simulink model "pfb_float_4k_sim"  (R2018b)
settings: Solver=VariableStepDiscrete  StartTime=0.0  StopTime=8192*2^2  RelTol=1e-3
undefined symbols (not defined in the file): fft_stages
libraries: xbsIndex_r4 x16102, casper_library_misc x27, casper_library_delays x15, casper_library_sources x4, xbsReplacements_r4 x3, xps_library x1
(part 8 of 133)

== system pfb_float_4k_sim/pfb_of ==
  out_reg : Inport
  assert_reg : xbsIndex_r4/Assert  assert_type=on  type_source=Explicitly  gui_display_data_type=Fixed-point  arith_type=Unsigned  n_bits=2  bin_pt=0  preci_type=Single  exp_width=8  frac_width=24  assert_rate=off  rate_source=Explicitly  period=1  output_port=on  block_type=assert
  cast_gw : xbsIndex_r4/Convert  gui_display_data_type=Fixed-point  arith_type=Unsigned  n_bits=32  bin_pt=0  float_type=Single  exp_bits=8  fraction_bits=24  quantization=Truncate  overflow=Wrap  en=off  latency=1  pipeline=off  dbl_ovrd=off  block_type=convert
  io_delay : xbsIndex_r4/Delay  rst=off  infoeditControl=Selection of Reset will increase slice count due to use of real FFs and instead of SRLs  en=off  latency=0  reg_retiming=on  dbl_ovrd=off  block_type=delay
  pfb_float_4k_sim_pfb_of_user_data_in : xbsIndex_r4/Gateway Out  inherit_from_input=off  hdl_port=on  rows=1  columns=1  UseAsDAC=off  DACChannel='1'  interface=None  automatic_assignment=on  address_offset=hex2dec('00')  intf_name=''  intf_description=''  timing_constraint=None  locs_specified=off  LOCs={}  io_standard={}  block_type=gatewayout
  reint1 : xbsIndex_r4/Reinterpret  force_arith_type=on  arith_type=Unsigned  force_bin_pt=on  bin_pt=0  block_type=reinterpret
  sim_out : Outport
  wires:
  io_delay/1 -> cast_gw/1
  cast_gw/1 -> pfb_float_4k_sim_pfb_of_user_data_in/1
  pfb_float_4k_sim_pfb_of_user_data_in/1 -> sim_out/1
  out_reg/1 -> assert_reg/1
  assert_reg/1 -> reint1/1
  reint1/1 -> io_delay/1

== system pfb_float_4k_sim/pfbin_sync_count ==
  out_reg : Inport
  assert_reg : xbsIndex_r4/Assert  assert_type=on  type_source=Explicitly  gui_display_data_type=Fixed-point  arith_type=Unsigned  n_bits=32  bin_pt=0  preci_type=Single  exp_width=8  frac_width=24  assert_rate=off  rate_source=Explicitly  period=1  output_port=on  block_type=assert
  cast_gw : xbsIndex_r4/Convert  gui_display_data_type=Fixed-point  arith_type=Unsigned  n_bits=32  bin_pt=0  float_type=Single  exp_bits=8  fraction_bits=24  quantization=Truncate  overflow=Wrap  en=off  latency=1  pipeline=off  dbl_ovrd=off  block_type=convert
  io_delay : xbsIndex_r4/Delay  rst=off  infoeditControl=Selection of Reset will increase slice count due to use of real FFs and instead of SRLs  en=off  latency=0  reg_retiming=on  dbl_ovrd=off  block_type=delay
  pfb_float_4k_sim_pfbin_sync_count_user_data_in : xbsIndex_r4/Gateway Out  inherit_from_input=off  hdl_port=on  rows=1  columns=1  UseAsDAC=off  DACChannel='1'  interface=None  automatic_assignment=on  address_offset=hex2dec('00')  intf_name=''  intf_description=''  timing_constraint=None  locs_specified=off  LOCs={}  io_standard={}  block_type=gatewayout
  reint1 : xbsIndex_r4/Reinterpret  force_arith_type=on  arith_type=Unsigned  force_bin_pt=on  bin_pt=0  block_type=reinterpret
  sim_out : Outport
  wires:
  io_delay/1 -> cast_gw/1
  cast_gw/1 -> pfb_float_4k_sim_pfbin_sync_count_user_data_in/1
  pfb_float_4k_sim_pfbin_sync_count_user_data_in/1 -> sim_out/1
  out_reg/1 -> assert_reg/1
  assert_reg/1 -> reint1/1
  reint1/1 -> io_delay/1

== system pfb_float_4k_sim/pfbout_sync_count ==
  out_reg : Inport
  assert_reg : xbsIndex_r4/Assert  assert_type=on  type_source=Explicitly  gui_display_data_type=Fixed-point  arith_type=Unsigned  n_bits=32  bin_pt=0  preci_type=Single  exp_width=8  frac_width=24  assert_rate=off  rate_source=Explicitly  period=1  output_port=on  block_type=assert
  cast_gw : xbsIndex_r4/Convert  gui_display_data_type=Fixed-point  arith_type=Unsigned  n_bits=32  bin_pt=0  float_type=Single  exp_bits=8  fraction_bits=24  quantization=Truncate  overflow=Wrap  en=off  latency=1  pipeline=off  dbl_ovrd=off  block_type=convert
  io_delay : xbsIndex_r4/Delay  rst=off  infoeditControl=Selection of Reset will increase slice count due to use of real FFs and instead of SRLs  en=off  latency=0  reg_retiming=on  dbl_ovrd=off  block_type=delay
  pfb_float_4k_sim_pfbout_sync_count_user_data_in : xbsIndex_r4/Gateway Out  inherit_from_input=off  hdl_port=on  rows=1  columns=1  UseAsDAC=off  DACChannel='1'  interface=None  automatic_assignment=on  address_offset=hex2dec('00')  intf_name=''  intf_description=''  timing_constraint=None  locs_specified=off  LOCs={}  io_standard={}  block_type=gatewayout
  reint1 : xbsIndex_r4/Reinterpret  force_arith_type=on  arith_type=Unsigned  force_bin_pt=on  bin_pt=0  block_type=reinterpret
  sim_out : Outport
  wires:
  io_delay/1 -> cast_gw/1
  cast_gw/1 -> pfb_float_4k_sim_pfbout_sync_count_user_data_in/1
  pfb_float_4k_sim_pfbout_sync_count_user_data_in/1 -> sim_out/1
  out_reg/1 -> assert_reg/1
  assert_reg/1 -> reint1/1
  reint1/1 -> io_delay/1

== system pfb_float_4k_sim/pipeline1 ==
  d : Inport
  Register0 : xbsIndex_r4/Register  init=0  rst=off  en=off  dbl_ovrd=off  block_type=register
      settings: block_version=11.4
  Register1 : xbsIndex_r4/Register  init=0  rst=off  en=off  dbl_ovrd=off  block_type=register
      settings: block_version=11.4
  q : Outport
  wires:
  Register0/1 -> Register1/1
  d/1 -> Register0/1
  Register1/1 -> q/1

== system pfb_float_4k_sim/pipeline10 ==
  d : Inport
  Register0 : xbsIndex_r4/Register  init=0  rst=off  en=off  dbl_ovrd=off  block_type=register
      settings: block_version=11.4
  Register1 : xbsIndex_r4/Register  init=0  rst=off  en=off  dbl_ovrd=off  block_type=register
      settings: block_version=11.4
  q : Outport
  wires:
  Register0/1 -> Register1/1
  d/1 -> Register0/1
  Register1/1 -> q/1

== system pfb_float_4k_sim/pipeline11 ==
  d : Inport
  Register0 : xbsIndex_r4/Register  init=0  rst=off  en=off  dbl_ovrd=off  block_type=register
      settings: block_version=11.4
  q : Outport
  wires:
  d/1 -> Register0/1
  Register0/1 -> q/1

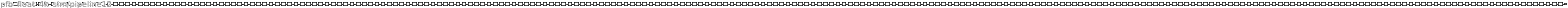
== system pfb_float_4k_sim/pipeline12 ==
  d : Inport
  Register{0..249} (250 alike) : xbsIndex_r4/Register  init=0  rst=off  en=off  dbl_ovrd=off  block_type=register
      Register0 settings: block_version=11.4
      Register1 settings: block_version=11.4
      Register2 settings: block_version=11.4
  q : Outport
  wires:
  Register0/1 -> Register1/1
  Register1/1 -> Register2/1
  Register2/1 -> Register3/1
  Register3/1 -> Register4/1
  Register4/1 -> Register5/1
  Register5/1 -> Register6/1
  Register6/1 -> Register7/1
  Register7/1 -> Register8/1
  Register8/1 -> Register9/1
  Register9/1 -> Register10/1
  Register10/1 -> Register11/1
  Register11/1 -> Register12/1
  Register12/1 -> Register13/1
  Register13/1 -> Register14/1
  Register14/1 -> Register15/1
  Register15/1 -> Register16/1
  Register16/1 -> Register17/1
  Register17/1 -> Register18/1
  Register18/1 -> Register19/1
  Register19/1 -> Register20/1
  Register20/1 -> Register21/1
  Register21/1 -> Register22/1
  Register22/1 -> Register23/1
  Register23/1 -> Register24/1
  Register24/1 -> Register25/1
  Register25/1 -> Register26/1
  Register26/1 -> Register27/1
  Register27/1 -> Register28/1
  Register28/1 -> Register29/1
  Register29/1 -> Register30/1
  Register30/1 -> Register31/1
  Register31/1 -> Register32/1
  Register32/1 -> Register33/1
  Register33/1 -> Register34/1
  Register34/1 -> Register35/1
  Register35/1 -> Register36/1
  Register36/1 -> Register37/1
  Register37/1 -> Register38/1
  Register38/1 -> Register39/1
  Register39/1 -> Register40/1
  Register40/1 -> Register41/1
  Register41/1 -> Register42/1
  Register42/1 -> Register43/1
  Register43/1 -> Register44/1
  Register44/1 -> Register45/1
  Register45/1 -> Register46/1
  Register46/1 -> Register47/1
  Register47/1 -> Register48/1
  Register48/1 -> Register49/1
  Register49/1 -> Register50/1
  Register50/1 -> Register51/1
  Register51/1 -> Register52/1
  Register52/1 -> Register53/1
  Register53/1 -> Register54/1
  Register54/1 -> Register55/1
  Register55/1 -> Register56/1
  Register56/1 -> Register57/1
  Register57/1 -> Register58/1
  Register58/1 -> Register59/1
  Register59/1 -> Register60/1
  Register60/1 -> Register61/1
  Register61/1 -> Register62/1
  Register62/1 -> Register63/1
  Register63/1 -> Register64/1
  Register64/1 -> Register65/1
  Register65/1 -> Register66/1
  Register66/1 -> Register67/1
  Register67/1 -> Register68/1
  Register68/1 -> Register69/1
  Register69/1 -> Register70/1
  Register70/1 -> Register71/1
  Register71/1 -> Register72/1
  Register72/1 -> Register73/1
  Register73/1 -> Register74/1
  Register74/1 -> Register75/1
  Register75/1 -> Register76/1
  Register76/1 -> Register77/1
  Register77/1 -> Register78/1
  Register78/1 -> Register79/1
  Register79/1 -> Register80/1
  Register80/1 -> Register81/1
  Register81/1 -> Register82/1
  Register82/1 -> Register83/1
  Register83/1 -> Register84/1
  Register84/1 -> Register85/1
  Register85/1 -> Register86/1
  Register86/1 -> Register87/1
  Register87/1 -> Register88/1
  Register88/1 -> Register89/1
  Register89/1 -> Register90/1
  Register90/1 -> Register91/1
  Register91/1 -> Register92/1
  Register92/1 -> Register93/1
  Register93/1 -> Register94/1
  Register94/1 -> Register95/1
  Register95/1 -> Register96/1
  Register96/1 -> Register97/1
  Register97/1 -> Register98/1
  Register98/1 -> Register99/1
  Register99/1 -> Register100/1
  Register100/1 -> Register101/1
  Register101/1 -> Register102/1
  Register102/1 -> Register103/1
  Register103/1 -> Register104/1
  Register104/1 -> Register105/1
  Register105/1 -> Register106/1
  Register106/1 -> Register107/1
  Register107/1 -> Register108/1
  Register108/1 -> Register109/1
  Register109/1 -> Register110/1
  Register110/1 -> Register111/1
  Register111/1 -> Register112/1
  Register112/1 -> Register113/1
  Register113/1 -> Register114/1
  Register114/1 -> Register115/1
  Register115/1 -> Register116/1
  Register116/1 -> Register117/1
  Register117/1 -> Register118/1
  Register118/1 -> Register119/1
  Register119/1 -> Register120/1
  Register120/1 -> Register121/1
  Register121/1 -> Register122/1
  Register122/1 -> Register123/1
  Register123/1 -> Register124/1
  Register124/1 -> Register125/1
  Register125/1 -> Register126/1
  Register126/1 -> Register127/1
  Register127/1 -> Register128/1
  Register128/1 -> Register129/1
  Register129/1 -> Register130/1
  Register130/1 -> Register131/1
  Register131/1 -> Register132/1
  Register132/1 -> Register133/1
  Register133/1 -> Register134/1
  Register134/1 -> Register135/1
  Register135/1 -> Register136/1
  Register136/1 -> Register137/1
  Register137/1 -> Register138/1
  Register138/1 -> Register139/1
  Register139/1 -> Register140/1
  Register140/1 -> Register141/1
  Register141/1 -> Register142/1
  Register142/1 -> Register143/1
  Register143/1 -> Register144/1
  Register144/1 -> Register145/1
  Register145/1 -> Register146/1
  Register146/1 -> Register147/1
  Register147/1 -> Register148/1
  Register148/1 -> Register149/1
  Register149/1 -> Register150/1
  Register150/1 -> Register151/1
  Register151/1 -> Register152/1
  Register152/1 -> Register153/1
  Register153/1 -> Register154/1
  Register154/1 -> Register155/1
  Register155/1 -> Register156/1
  Register156/1 -> Register157/1
  Register157/1 -> Register158/1
  Register158/1 -> Register159/1
  Register159/1 -> Register160/1
  Register160/1 -> Register161/1
  Register161/1 -> Register162/1
  Register162/1 -> Register163/1
  Register163/1 -> Register164/1
  Register164/1 -> Register165/1
  Register165/1 -> Register166/1
  Register166/1 -> Register167/1
  Register167/1 -> Register168/1
  Register168/1 -> Register169/1
  Register169/1 -> Register170/1
  Register170/1 -> Register171/1
  Register171/1 -> Register172/1
  Register172/1 -> Register173/1
  Register173/1 -> Register174/1
  Register174/1 -> Register175/1
  Register175/1 -> Register176/1
  Register176/1 -> Register177/1
  Register177/1 -> Register178/1
  Register178/1 -> Register179/1
  Register179/1 -> Register180/1
  Register180/1 -> Register181/1
  Register181/1 -> Register182/1
  Register182/1 -> Register183/1
  Register183/1 -> Register184/1
  Register184/1 -> Register185/1
  Register185/1 -> Register186/1
  Register186/1 -> Register187/1
  Register187/1 -> Register188/1
  Register188/1 -> Register189/1
  Register189/1 -> Register190/1
  Register190/1 -> Register191/1
  Register191/1 -> Register192/1
  Register192/1 -> Register193/1
  Register193/1 -> Register194/1
  Register194/1 -> Register195/1
  Register195/1 -> Register196/1
  Register196/1 -> Register197/1
  Register197/1 -> Register198/1
  Register198/1 -> Register199/1
  Register199/1 -> Register200/1
  Register200/1 -> Register201/1
  Register201/1 -> Register202/1
  Register202/1 -> Register203/1
  Register203/1 -> Register204/1
  Register204/1 -> Register205/1
  Register205/1 -> Register206/1
  Register206/1 -> Register207/1
  Register207/1 -> Register208/1
  Register208/1 -> Register209/1
  Register209/1 -> Register210/1
  Register210/1 -> Register211/1
  Register211/1 -> Register212/1
  Register212/1 -> Register213/1
  Register213/1 -> Register214/1
  Register214/1 -> Register215/1
  Register215/1 -> Register216/1
  Register216/1 -> Register217/1
  Register217/1 -> Register218/1
  Register218/1 -> Register219/1
  Register219/1 -> Register220/1
  Register220/1 -> Register221/1
  Register221/1 -> Register222/1
  Register222/1 -> Register223/1
  Register223/1 -> Register224/1
  Register224/1 -> Register225/1
  Register225/1 -> Register226/1
  Register226/1 -> Register227/1
  Register227/1 -> Register228/1
  Register228/1 -> Register229/1
  Register229/1 -> Register230/1
  Register230/1 -> Register231/1
  Register231/1 -> Register232/1
  Register232/1 -> Register233/1
  Register233/1 -> Register234/1
  Register234/1 -> Register235/1
  Register235/1 -> Register236/1
  Register236/1 -> Register237/1
  Register237/1 -> Register238/1
  Register238/1 -> Register239/1
  Register239/1 -> Register240/1
  Register240/1 -> Register241/1
  Register241/1 -> Register242/1
  Register242/1 -> Register243/1
  Register243/1 -> Register244/1
  Register244/1 -> Register245/1
  Register245/1 -> Register246/1
  Register246/1 -> Register247/1
  Register247/1 -> Register248/1
  Register248/1 -> Register249/1
  d/1 -> Register0/1
  Register249/1 -> q/1

== system pfb_float_4k_sim/pipeline13 ==
  d : Inport
  Register0 : xbsIndex_r4/Register  init=0  rst=off  en=off  dbl_ovrd=off  block_type=register
      settings: block_version=11.4
  Register1 : xbsIndex_r4/Register  init=0  rst=off  en=off  dbl_ovrd=off  block_type=register
      settings: block_version=11.4
  q : Outport
  wires:
  Register0/1 -> Register1/1
  d/1 -> Register0/1
  Register1/1 -> q/1

== system pfb_float_4k_sim/pipeline14 ==
  d : Inport
  Register0 : xbsIndex_r4/Register  init=0  rst=off  en=off  dbl_ovrd=off  block_type=register
      settings: block_version=11.4
  Register1 : xbsIndex_r4/Register  init=0  rst=off  en=off  dbl_ovrd=off  block_type=register
      settings: block_version=11.4
  q : Outport
  wires:
  Register0/1 -> Register1/1
  d/1 -> Register0/1
  Register1/1 -> q/1

== system pfb_float_4k_sim/pipeline15 ==
  d : Inport
  Register0 : xbsIndex_r4/Register  init=0  rst=off  en=off  dbl_ovrd=off  block_type=register
      settings: block_version=11.4
  q : Outport
  wires:
  d/1 -> Register0/1
  Register0/1 -> q/1

== system pfb_float_4k_sim/pipeline16 ==
  d : Inport
  Register{0..3} (4 alike) : xbsIndex_r4/Register  init=0  rst=off  en=off  dbl_ovrd=off  block_type=register
      Register0 settings: block_version=11.4
      Register1 settings: block_version=11.4
      Register2 settings: block_version=11.4
  q : Outport
  wires:
  Register0/1 -> Register1/1
  Register1/1 -> Register2/1
  Register2/1 -> Register3/1
  d/1 -> Register0/1
  Register3/1 -> q/1

== system pfb_float_4k_sim/pipeline17 ==
  d : Inport
  Register0 : xbsIndex_r4/Register  init=0  rst=off  en=off  dbl_ovrd=off  block_type=register
      settings: block_version=11.4
  Register1 : xbsIndex_r4/Register  init=0  rst=off  en=off  dbl_ovrd=off  block_type=register
      settings: block_version=11.4
  q : Outport
  wires:
  Register0/1 -> Register1/1
  d/1 -> Register0/1
  Register1/1 -> q/1

== system pfb_float_4k_sim/pipeline18 ==
  d : Inport
  Register0 : xbsIndex_r4/Register  init=0  rst=off  en=off  dbl_ovrd=off  block_type=register
      settings: block_version=11.4
  Register1 : xbsIndex_r4/Register  init=0  rst=off  en=off  dbl_ovrd=off  block_type=register
      settings: block_version=11.4
  q : Outport
  wires:
  Register0/1 -> Register1/1
  d/1 -> Register0/1
  Register1/1 -> q/1

== system pfb_float_4k_sim/pipeline180 ==
  d : Inport
  Register0 : xbsIndex_r4/Register  init=0  rst=off  en=off  dbl_ovrd=off  block_type=register
      settings: block_version=11.4
  Register1 : xbsIndex_r4/Register  init=0  rst=off  en=off  dbl_ovrd=off  block_type=register
      settings: block_version=11.4
  q : Outport
  wires:
  Register0/1 -> Register1/1
  d/1 -> Register0/1
  Register1/1 -> q/1

== system pfb_float_4k_sim/pipeline181 ==
  d : Inport
  Register0 : xbsIndex_r4/Register  init=0  rst=off  en=off  dbl_ovrd=off  block_type=register
      settings: block_version=11.4
  Register1 : xbsIndex_r4/Register  init=0  rst=off  en=off  dbl_ovrd=off  block_type=register
      settings: block_version=11.4
  q : Outport
  wires:
  Register0/1 -> Register1/1
  d/1 -> Register0/1
  Register1/1 -> q/1

== system pfb_float_4k_sim/pipeline182 ==
  d : Inport
  Register0 : xbsIndex_r4/Register  init=0  rst=off  en=off  dbl_ovrd=off  block_type=register
      settings: block_version=11.4
  Register1 : xbsIndex_r4/Register  init=0  rst=off  en=off  dbl_ovrd=off  block_type=register
      settings: block_version=11.4
  q : Outport
  wires:
  Register0/1 -> Register1/1
  d/1 -> Register0/1
  Register1/1 -> q/1

== system pfb_float_4k_sim/pipeline19 ==
  d : Inport
  Register0 : xbsIndex_r4/Register  init=0  rst=off  en=off  dbl_ovrd=off  block_type=register
      settings: block_version=11.4
  Register1 : xbsIndex_r4/Register  init=0  rst=off  en=off  dbl_ovrd=off  block_type=register
      settings: block_version=11.4
  q : Outport
  wires:
  Register0/1 -> Register1/1
  d/1 -> Register0/1
  Register1/1 -> q/1

== system pfb_float_4k_sim/pipeline198 ==
  d : Inport
  Register0 : xbsIndex_r4/Register  init=0  rst=off  en=off  dbl_ovrd=off  block_type=register
      settings: block_version=11.4
  Register1 : xbsIndex_r4/Register  init=0  rst=off  en=off  dbl_ovrd=off  block_type=register
      settings: block_version=11.4
  q : Outport
  wires:
  Register0/1 -> Register1/1
  d/1 -> Register0/1
  Register1/1 -> q/1

== system pfb_float_4k_sim/pipeline2 ==
  d : Inport
  Register0 : xbsIndex_r4/Register  init=0  rst=off  en=off  dbl_ovrd=off  block_type=register
      settings: block_version=11.4
  Register1 : xbsIndex_r4/Register  init=0  rst=off  en=off  dbl_ovrd=off  block_type=register
      settings: block_version=11.4
  q : Outport
  wires:
  Register0/1 -> Register1/1
  d/1 -> Register0/1
  Register1/1 -> q/1

== system pfb_float_4k_sim/pipeline20 ==
  d : Inport
  Register0 : xbsIndex_r4/Register  init=0  rst=off  en=off  dbl_ovrd=off  block_type=register
      settings: block_version=11.4
  Register1 : xbsIndex_r4/Register  init=0  rst=off  en=off  dbl_ovrd=off  block_type=register
      settings: block_version=11.4
  q : Outport
  wires:
  Register0/1 -> Register1/1
  d/1 -> Register0/1
  Register1/1 -> q/1

== system pfb_float_4k_sim/pipeline21 ==
  d : Inport
  Register0 : xbsIndex_r4/Register  init=0  rst=off  en=off  dbl_ovrd=off  block_type=register
      settings: block_version=11.4
  Register1 : xbsIndex_r4/Register  init=0  rst=off  en=off  dbl_ovrd=off  block_type=register
      settings: block_version=11.4
  q : Outport
  wires:
  Register0/1 -> Register1/1
  d/1 -> Register0/1
  Register1/1 -> q/1

== system pfb_float_4k_sim/pipeline22 ==
  d : Inport
  Register0 : xbsIndex_r4/Register  init=0  rst=off  en=off  dbl_ovrd=off  block_type=register
      settings: block_version=11.4
  Register1 : xbsIndex_r4/Register  init=0  rst=off  en=off  dbl_ovrd=off  block_type=register
      settings: block_version=11.4
  q : Outport
  wires:
  Register0/1 -> Register1/1
  d/1 -> Register0/1
  Register1/1 -> q/1

== system pfb_float_4k_sim/pipeline23 ==
  d : Inport
  Register0 : xbsIndex_r4/Register  init=0  rst=off  en=off  dbl_ovrd=off  block_type=register
      settings: block_version=11.4
  Register1 : xbsIndex_r4/Register  init=0  rst=off  en=off  dbl_ovrd=off  block_type=register
      settings: block_version=11.4
  q : Outport
  wires:
  Register0/1 -> Register1/1
  d/1 -> Register0/1
  Register1/1 -> q/1

== system pfb_float_4k_sim/pipeline24 ==
  d : Inport
  Register0 : xbsIndex_r4/Register  init=0  rst=off  en=off  dbl_ovrd=off  block_type=register
      settings: block_version=11.4
  Register1 : xbsIndex_r4/Register  init=0  rst=off  en=off  dbl_ovrd=off  block_type=register
      settings: block_version=11.4
  q : Outport
  wires:
  Register0/1 -> Register1/1
  d/1 -> Register0/1
  Register1/1 -> q/1

== system pfb_float_4k_sim/pipeline25 ==
  d : Inport
  Register0 : xbsIndex_r4/Register  init=0  rst=off  en=off  dbl_ovrd=off  block_type=register
      settings: block_version=11.4
  Register1 : xbsIndex_r4/Register  init=0  rst=off  en=off  dbl_ovrd=off  block_type=register
      settings: block_version=11.4
  q : Outport
  wires:
  Register0/1 -> Register1/1
  d/1 -> Register0/1
  Register1/1 -> q/1

== system pfb_float_4k_sim/pipeline26 ==
  d : Inport
  Register0 : xbsIndex_r4/Register  init=0  rst=off  en=off  dbl_ovrd=off  block_type=register
      settings: block_version=11.4
  Register1 : xbsIndex_r4/Register  init=0  rst=off  en=off  dbl_ovrd=off  block_type=register
      settings: block_version=11.4
  q : Outport
  wires:
  Register0/1 -> Register1/1
  d/1 -> Register0/1
  Register1/1 -> q/1

== system pfb_float_4k_sim/pipeline27 ==
  d : Inport
  Register0 : xbsIndex_r4/Register  init=0  rst=off  en=off  dbl_ovrd=off  block_type=register
      settings: block_version=11.4
  Register1 : xbsIndex_r4/Register  init=0  rst=off  en=off  dbl_ovrd=off  block_type=register
      settings: block_version=11.4
  q : Outport
  wires:
  Register0/1 -> Register1/1
  d/1 -> Register0/1
  Register1/1 -> q/1

== system pfb_float_4k_sim/pipeline28 ==
  d : Inport
  Register0 : xbsIndex_r4/Register  init=0  rst=off  en=off  dbl_ovrd=off  block_type=register
      settings: block_version=11.4
  Register1 : xbsIndex_r4/Register  init=0  rst=off  en=off  dbl_ovrd=off  block_type=register
      settings: block_version=11.4
  q : Outport
  wires:
  Register0/1 -> Register1/1
  d/1 -> Register0/1
  Register1/1 -> q/1

== system pfb_float_4k_sim/pipeline29 ==
  d : Inport
  Register0 : xbsIndex_r4/Register  init=0  rst=off  en=off  dbl_ovrd=off  block_type=register
      settings: block_version=11.4
  Register1 : xbsIndex_r4/Register  init=0  rst=off  en=off  dbl_ovrd=off  block_type=register
      settings: block_version=11.4
  q : Outport
  wires:
  Register0/1 -> Register1/1
  d/1 -> Register0/1
  Register1/1 -> q/1

== system pfb_float_4k_sim/pipeline3 ==
  d : Inport
  Register0 : xbsIndex_r4/Register  init=0  rst=off  en=off  dbl_ovrd=off  block_type=register
      settings: block_version=11.4
  Register1 : xbsIndex_r4/Register  init=0  rst=off  en=off  dbl_ovrd=off  block_type=register
      settings: block_version=11.4
  q : Outport
  wires:
  Register0/1 -> Register1/1
  d/1 -> Register0/1
  Register1/1 -> q/1

== system pfb_float_4k_sim/pipeline30 ==
  d : Inport
  Register0 : xbsIndex_r4/Register  init=0  rst=off  en=off  dbl_ovrd=off  block_type=register
      settings: block_version=11.4
  Register1 : xbsIndex_r4/Register  init=0  rst=off  en=off  dbl_ovrd=off  block_type=register
      settings: block_version=11.4
  q : Outport
  wires:
  Register0/1 -> Register1/1
  d/1 -> Register0/1
  Register1/1 -> q/1

== system pfb_float_4k_sim/pipeline31 ==
  d : Inport
  Register0 : xbsIndex_r4/Register  init=0  rst=off  en=off  dbl_ovrd=off  block_type=register
      settings: block_version=11.4
  Register1 : xbsIndex_r4/Register  init=0  rst=off  en=off  dbl_ovrd=off  block_type=register
      settings: block_version=11.4
  q : Outport
  wires:
  Register0/1 -> Register1/1
  d/1 -> Register0/1
  Register1/1 -> q/1

== system pfb_float_4k_sim/pipeline32 ==
  d : Inport
  Register0 : xbsIndex_r4/Register  init=0  rst=off  en=off  dbl_ovrd=off  block_type=register
      settings: block_version=11.4
  Register1 : xbsIndex_r4/Register  init=0  rst=off  en=off  dbl_ovrd=off  block_type=register
      settings: block_version=11.4
  q : Outport
  wires:
  Register0/1 -> Register1/1
  d/1 -> Register0/1
  Register1/1 -> q/1

== system pfb_float_4k_sim/pipeline33 ==
  d : Inport
  Register0 : xbsIndex_r4/Register  init=0  rst=off  en=off  dbl_ovrd=off  block_type=register
      settings: block_version=11.4
  Register1 : xbsIndex_r4/Register  init=0  rst=off  en=off  dbl_ovrd=off  block_type=register
      settings: block_version=11.4
  q : Outport
  wires:
  Register0/1 -> Register1/1
  d/1 -> Register0/1
  Register1/1 -> q/1

== system pfb_float_4k_sim/pipeline34 ==
  d : Inport
  Register0 : xbsIndex_r4/Register  init=0  rst=off  en=off  dbl_ovrd=off  block_type=register
      settings: block_version=11.4
  Register1 : xbsIndex_r4/Register  init=0  rst=off  en=off  dbl_ovrd=off  block_type=register
      settings: block_version=11.4
  q : Outport
  wires:
  Register0/1 -> Register1/1
  d/1 -> Register0/1
  Register1/1 -> q/1

== system pfb_float_4k_sim/pipeline35 ==
  d : Inport
  Register0 : xbsIndex_r4/Register  init=0  rst=off  en=off  dbl_ovrd=off  block_type=register
      settings: block_version=11.4
  Register1 : xbsIndex_r4/Register  init=0  rst=off  en=off  dbl_ovrd=off  block_type=register
      settings: block_version=11.4
  q : Outport
  wires:
  Register0/1 -> Register1/1
  d/1 -> Register0/1
  Register1/1 -> q/1

== system pfb_float_4k_sim/pipeline36 ==
  d : Inport
  Register0 : xbsIndex_r4/Register  init=0  rst=off  en=off  dbl_ovrd=off  block_type=register
      settings: block_version=11.4
  Register1 : xbsIndex_r4/Register  init=0  rst=off  en=off  dbl_ovrd=off  block_type=register
      settings: block_version=11.4
  q : Outport
  wires:
  Register0/1 -> Register1/1
  d/1 -> Register0/1
  Register1/1 -> q/1

== system pfb_float_4k_sim/pipeline37 ==
  d : Inport
  Register0 : xbsIndex_r4/Register  init=0  rst=off  en=off  dbl_ovrd=off  block_type=register
      settings: block_version=11.4
  Register1 : xbsIndex_r4/Register  init=0  rst=off  en=off  dbl_ovrd=off  block_type=register
      settings: block_version=11.4
  q : Outport
  wires:
  Register0/1 -> Register1/1
  d/1 -> Register0/1
  Register1/1 -> q/1

== system pfb_float_4k_sim/pipeline38 ==
  d : Inport
  Register0 : xbsIndex_r4/Register  init=0  rst=off  en=off  dbl_ovrd=off  block_type=register
      settings: block_version=11.4
  q : Outport
  wires:
  d/1 -> Register0/1
  Register0/1 -> q/1

== system pfb_float_4k_sim/pipeline39 ==
  d : Inport
  Register0 : xbsIndex_r4/Register  init=0  rst=off  en=off  dbl_ovrd=off  block_type=register
      settings: block_version=11.4
  q : Outport
  wires:
  d/1 -> Register0/1
  Register0/1 -> q/1

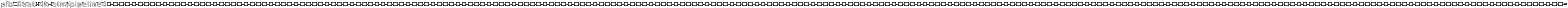
== system pfb_float_4k_sim/pipeline4 ==
  d : Inport
  Register{0..249} (250 alike) : xbsIndex_r4/Register  init=0  rst=off  en=off  dbl_ovrd=off  block_type=register
      Register0 settings: block_version=11.4
      Register1 settings: block_version=11.4
      Register2 settings: block_version=11.4
  q : Outport
  wires:
  Register0/1 -> Register1/1
  Register1/1 -> Register2/1
  Register2/1 -> Register3/1
  Register3/1 -> Register4/1
  Register4/1 -> Register5/1
  Register5/1 -> Register6/1
  Register6/1 -> Register7/1
  Register7/1 -> Register8/1
  Register8/1 -> Register9/1
  Register9/1 -> Register10/1
  Register10/1 -> Register11/1
  Register11/1 -> Register12/1
  Register12/1 -> Register13/1
  Register13/1 -> Register14/1
  Register14/1 -> Register15/1
  Register15/1 -> Register16/1
  Register16/1 -> Register17/1
  Register17/1 -> Register18/1
  Register18/1 -> Register19/1
  Register19/1 -> Register20/1
  Register20/1 -> Register21/1
  Register21/1 -> Register22/1
  Register22/1 -> Register23/1
  Register23/1 -> Register24/1
  Register24/1 -> Register25/1
  Register25/1 -> Register26/1
  Register26/1 -> Register27/1
  Register27/1 -> Register28/1
  Register28/1 -> Register29/1
  Register29/1 -> Register30/1
  Register30/1 -> Register31/1
  Register31/1 -> Register32/1
  Register32/1 -> Register33/1
  Register33/1 -> Register34/1
  Register34/1 -> Register35/1
  Register35/1 -> Register36/1
  Register36/1 -> Register37/1
  Register37/1 -> Register38/1
  Register38/1 -> Register39/1
  Register39/1 -> Register40/1
  Register40/1 -> Register41/1
  Register41/1 -> Register42/1
  Register42/1 -> Register43/1
  Register43/1 -> Register44/1
  Register44/1 -> Register45/1
  Register45/1 -> Register46/1
  Register46/1 -> Register47/1
  Register47/1 -> Register48/1
  Register48/1 -> Register49/1
  Register49/1 -> Register50/1
  Register50/1 -> Register51/1
  Register51/1 -> Register52/1
  Register52/1 -> Register53/1
  Register53/1 -> Register54/1
  Register54/1 -> Register55/1
  Register55/1 -> Register56/1
  Register56/1 -> Register57/1
  Register57/1 -> Register58/1
  Register58/1 -> Register59/1
  Register59/1 -> Register60/1
  Register60/1 -> Register61/1
  Register61/1 -> Register62/1
  Register62/1 -> Register63/1
  Register63/1 -> Register64/1
  Register64/1 -> Register65/1
  Register65/1 -> Register66/1
  Register66/1 -> Register67/1
  Register67/1 -> Register68/1
  Register68/1 -> Register69/1
  Register69/1 -> Register70/1
  Register70/1 -> Register71/1
  Register71/1 -> Register72/1
  Register72/1 -> Register73/1
  Register73/1 -> Register74/1
  Register74/1 -> Register75/1
  Register75/1 -> Register76/1
  Register76/1 -> Register77/1
  Register77/1 -> Register78/1
  Register78/1 -> Register79/1
  Register79/1 -> Register80/1
  Register80/1 -> Register81/1
  Register81/1 -> Register82/1
  Register82/1 -> Register83/1
  Register83/1 -> Register84/1
  Register84/1 -> Register85/1
  Register85/1 -> Register86/1
  Register86/1 -> Register87/1
  Register87/1 -> Register88/1
  Register88/1 -> Register89/1
  Register89/1 -> Register90/1
  Register90/1 -> Register91/1
  Register91/1 -> Register92/1
  Register92/1 -> Register93/1
  Register93/1 -> Register94/1
  Register94/1 -> Register95/1
  Register95/1 -> Register96/1
  Register96/1 -> Register97/1
  Register97/1 -> Register98/1
  Register98/1 -> Register99/1
  Register99/1 -> Register100/1
  Register100/1 -> Register101/1
  Register101/1 -> Register102/1
  Register102/1 -> Register103/1
  Register103/1 -> Register104/1
  Register104/1 -> Register105/1
  Register105/1 -> Register106/1
  Register106/1 -> Register107/1
  Register107/1 -> Register108/1
  Register108/1 -> Register109/1
  Register109/1 -> Register110/1
  Register110/1 -> Register111/1
  Register111/1 -> Register112/1
  Register112/1 -> Register113/1
  Register113/1 -> Register114/1
  Register114/1 -> Register115/1
  Register115/1 -> Register116/1
  Register116/1 -> Register117/1
  Register117/1 -> Register118/1
  Register118/1 -> Register119/1
  Register119/1 -> Register120/1
  Register120/1 -> Register121/1
  Register121/1 -> Register122/1
  Register122/1 -> Register123/1
  Register123/1 -> Register124/1
  Register124/1 -> Register125/1
  Register125/1 -> Register126/1
  Register126/1 -> Register127/1
  Register127/1 -> Register128/1
  Register128/1 -> Register129/1
  Register129/1 -> Register130/1
  Register130/1 -> Register131/1
  Register131/1 -> Register132/1
  Register132/1 -> Register133/1
  Register133/1 -> Register134/1
  Register134/1 -> Register135/1
  Register135/1 -> Register136/1
  Register136/1 -> Register137/1
  Register137/1 -> Register138/1
  Register138/1 -> Register139/1
  Register139/1 -> Register140/1
  Register140/1 -> Register141/1
  Register141/1 -> Register142/1
  Register142/1 -> Register143/1
  Register143/1 -> Register144/1
  Register144/1 -> Register145/1
  Register145/1 -> Register146/1
  Register146/1 -> Register147/1
  Register147/1 -> Register148/1
  Register148/1 -> Register149/1
  Register149/1 -> Register150/1
  Register150/1 -> Register151/1
  Register151/1 -> Register152/1
  Register152/1 -> Register153/1
  Register153/1 -> Register154/1
  Register154/1 -> Register155/1
  Register155/1 -> Register156/1
  Register156/1 -> Register157/1
  Register157/1 -> Register158/1
  Register158/1 -> Register159/1
  Register159/1 -> Register160/1
  Register160/1 -> Register161/1
  Register161/1 -> Register162/1
  Register162/1 -> Register163/1
  Register163/1 -> Register164/1
  Register164/1 -> Register165/1
  Register165/1 -> Register166/1
  Register166/1 -> Register167/1
  Register167/1 -> Register168/1
  Register168/1 -> Register169/1
  Register169/1 -> Register170/1
  Register170/1 -> Register171/1
  Register171/1 -> Register172/1
  Register172/1 -> Register173/1
  Register173/1 -> Register174/1
  Register174/1 -> Register175/1
  Register175/1 -> Register176/1
  Register176/1 -> Register177/1
  Register177/1 -> Register178/1
  Register178/1 -> Register179/1
  Register179/1 -> Register180/1
  Register180/1 -> Register181/1
  Register181/1 -> Register182/1
  Register182/1 -> Register183/1
  Register183/1 -> Register184/1
  Register184/1 -> Register185/1
  Register185/1 -> Register186/1
  Register186/1 -> Register187/1
  Register187/1 -> Register188/1
  Register188/1 -> Register189/1
  Register189/1 -> Register190/1
  Register190/1 -> Register191/1
  Register191/1 -> Register192/1
  Register192/1 -> Register193/1
  Register193/1 -> Register194/1
  Register194/1 -> Register195/1
  Register195/1 -> Register196/1
  Register196/1 -> Register197/1
  Register197/1 -> Register198/1
  Register198/1 -> Register199/1
  Register199/1 -> Register200/1
  Register200/1 -> Register201/1
  Register201/1 -> Register202/1
  Register202/1 -> Register203/1
  Register203/1 -> Register204/1
  Register204/1 -> Register205/1
  Register205/1 -> Register206/1
  Register206/1 -> Register207/1
  Register207/1 -> Register208/1
  Register208/1 -> Register209/1
  Register209/1 -> Register210/1
  Register210/1 -> Register211/1
  Register211/1 -> Register212/1
  Register212/1 -> Register213/1
  Register213/1 -> Register214/1
  Register214/1 -> Register215/1
  Register215/1 -> Register216/1
  Register216/1 -> Register217/1
  Register217/1 -> Register218/1
  Register218/1 -> Register219/1
  Register219/1 -> Register220/1
  Register220/1 -> Register221/1
  Register221/1 -> Register222/1
  Register222/1 -> Register223/1
  Register223/1 -> Register224/1
  Register224/1 -> Register225/1
  Register225/1 -> Register226/1
  Register226/1 -> Register227/1
  Register227/1 -> Register228/1
  Register228/1 -> Register229/1
  Register229/1 -> Register230/1
  Register230/1 -> Register231/1
  Register231/1 -> Register232/1
  Register232/1 -> Register233/1
  Register233/1 -> Register234/1
  Register234/1 -> Register235/1
  Register235/1 -> Register236/1
  Register236/1 -> Register237/1
  Register237/1 -> Register238/1
  Register238/1 -> Register239/1
  Register239/1 -> Register240/1
  Register240/1 -> Register241/1
  Register241/1 -> Register242/1
  Register242/1 -> Register243/1
  Register243/1 -> Register244/1
  Register244/1 -> Register245/1
  Register245/1 -> Register246/1
  Register246/1 -> Register247/1
  Register247/1 -> Register248/1
  Register248/1 -> Register249/1
  d/1 -> Register0/1
  Register249/1 -> q/1

== system pfb_float_4k_sim/pipeline40 ==
  d : Inport
  Register0 : xbsIndex_r4/Register  init=0  rst=off  en=off  dbl_ovrd=off  block_type=register
      settings: block_version=11.4
  q : Outport
  wires:
  d/1 -> Register0/1
  Register0/1 -> q/1

== system pfb_float_4k_sim/pipeline41 ==
  d : Inport
  Register0 : xbsIndex_r4/Register  init=0  rst=off  en=off  dbl_ovrd=off  block_type=register
      settings: block_version=11.4
  Register1 : xbsIndex_r4/Register  init=0  rst=off  en=off  dbl_ovrd=off  block_type=register
      settings: block_version=11.4
  q : Outport
  wires:
  Register0/1 -> Register1/1
  d/1 -> Register0/1
  Register1/1 -> q/1

== system pfb_float_4k_sim/pipeline42 ==
  d : Inport
  Register0 : xbsIndex_r4/Register  init=0  rst=off  en=off  dbl_ovrd=off  block_type=register
      settings: block_version=11.4
  q : Outport
  wires:
  d/1 -> Register0/1
  Register0/1 -> q/1

== system pfb_float_4k_sim/pipeline43 ==
  d : Inport
  Register0 : xbsIndex_r4/Register  init=0  rst=off  en=off  dbl_ovrd=off  block_type=register
      settings: block_version=11.4
  q : Outport
  wires:
  d/1 -> Register0/1
  Register0/1 -> q/1

== system pfb_float_4k_sim/pipeline44 ==
  d : Inport
  Register0 : xbsIndex_r4/Register  init=0  rst=off  en=off  dbl_ovrd=off  block_type=register
      settings: block_version=11.4
  Register1 : xbsIndex_r4/Register  init=0  rst=off  en=off  dbl_ovrd=off  block_type=register
      settings: block_version=11.4
  q : Outport
  wires:
  Register0/1 -> Register1/1
  d/1 -> Register0/1
  Register1/1 -> q/1

== system pfb_float_4k_sim/pipeline45 ==
  d : Inport
  Register0 : xbsIndex_r4/Register  init=0  rst=off  en=off  dbl_ovrd=off  block_type=register
      settings: block_version=11.4
  Register1 : xbsIndex_r4/Register  init=0  rst=off  en=off  dbl_ovrd=off  block_type=register
      settings: block_version=11.4
  q : Outport
  wires:
  Register0/1 -> Register1/1
  d/1 -> Register0/1
  Register1/1 -> q/1

== system pfb_float_4k_sim/pipeline46 ==
  d : Inport
  Register0 : xbsIndex_r4/Register  init=0  rst=off  en=off  dbl_ovrd=off  block_type=register
      settings: block_version=11.4
  q : Outport
  wires:
  d/1 -> Register0/1
  Register0/1 -> q/1

== system pfb_float_4k_sim/pipeline47 ==
  d : Inport
  Register0 : xbsIndex_r4/Register  init=0  rst=off  en=off  dbl_ovrd=off  block_type=register
      settings: block_version=11.4
  Register1 : xbsIndex_r4/Register  init=0  rst=off  en=off  dbl_ovrd=off  block_type=register
      settings: block_version=11.4
  q : Outport
  wires:
  Register0/1 -> Register1/1
  d/1 -> Register0/1
  Register1/1 -> q/1

== system pfb_float_4k_sim/pipeline48 ==
  d : Inport
  Register0 : xbsIndex_r4/Register  init=0  rst=off  en=off  dbl_ovrd=off  block_type=register
      settings: block_version=11.4
  Register1 : xbsIndex_r4/Register  init=0  rst=off  en=off  dbl_ovrd=off  block_type=register
      settings: block_version=11.4
  q : Outport
  wires:
  Register0/1 -> Register1/1
  d/1 -> Register0/1
  Register1/1 -> q/1

== system pfb_float_4k_sim/pipeline49 ==
  d : Inport
  Register0 : xbsIndex_r4/Register  init=0  rst=off  en=off  dbl_ovrd=off  block_type=register
      settings: block_version=11.4
  Register1 : xbsIndex_r4/Register  init=0  rst=off  en=off  dbl_ovrd=off  block_type=register
      settings: block_version=11.4
  q : Outport
  wires:
  Register0/1 -> Register1/1
  d/1 -> Register0/1
  Register1/1 -> q/1

== system pfb_float_4k_sim/pipeline5 ==
  d : Inport
  Register0 : xbsIndex_r4/Register  init=0  rst=off  en=off  dbl_ovrd=off  block_type=register
      settings: block_version=11.4
  Register1 : xbsIndex_r4/Register  init=0  rst=off  en=off  dbl_ovrd=off  block_type=register
      settings: block_version=11.4
  q : Outport
  wires:
  Register0/1 -> Register1/1
  d/1 -> Register0/1
  Register1/1 -> q/1

== system pfb_float_4k_sim/pipeline50 ==
  d : Inport
  Register0 : xbsIndex_r4/Register  init=0  rst=off  en=off  dbl_ovrd=off  block_type=register
      settings: block_version=11.4
  Register1 : xbsIndex_r4/Register  init=0  rst=off  en=off  dbl_ovrd=off  block_type=register
      settings: block_version=11.4
  q : Outport
  wires:
  Register0/1 -> Register1/1
  d/1 -> Register0/1
  Register1/1 -> q/1

== system pfb_float_4k_sim/pipeline51 ==
  d : Inport
  Register0 : xbsIndex_r4/Register  init=0  rst=off  en=off  dbl_ovrd=off  block_type=register
      settings: block_version=11.4
  Register1 : xbsIndex_r4/Register  init=0  rst=off  en=off  dbl_ovrd=off  block_type=register
      settings: block_version=11.4
  q : Outport
  wires:
  Register0/1 -> Register1/1
  d/1 -> Register0/1
  Register1/1 -> q/1

== system pfb_float_4k_sim/pipeline52 ==
  d : Inport
  Register0 : xbsIndex_r4/Register  init=0  rst=off  en=off  dbl_ovrd=off  block_type=register
      settings: block_version=11.4
  Register1 : xbsIndex_r4/Register  init=0  rst=off  en=off  dbl_ovrd=off  block_type=register
      settings: block_version=11.4
  q : Outport
  wires:
  Register0/1 -> Register1/1
  d/1 -> Register0/1
  Register1/1 -> q/1

== system pfb_float_4k_sim/pipeline53 ==
  d : Inport
  Register0 : xbsIndex_r4/Register  init=0  rst=off  en=off  dbl_ovrd=off  block_type=register
      settings: block_version=11.4
  q : Outport
  wires:
  d/1 -> Register0/1
  Register0/1 -> q/1

== system pfb_float_4k_sim/pipeline54 ==
  d : Inport
  Register0 : xbsIndex_r4/Register  init=0  rst=off  en=off  dbl_ovrd=off  block_type=register
      settings: block_version=11.4
  Register1 : xbsIndex_r4/Register  init=0  rst=off  en=off  dbl_ovrd=off  block_type=register
      settings: block_version=11.4
  q : Outport
  wires:
  Register0/1 -> Register1/1
  d/1 -> Register0/1
  Register1/1 -> q/1

== system pfb_float_4k_sim/pipeline55 ==
  d : Inport
  Register{0..3} (4 alike) : xbsIndex_r4/Register  init=0  rst=off  en=off  dbl_ovrd=off  block_type=register
      Register0 settings: block_version=11.4
      Register1 settings: block_version=11.4
      Register2 settings: block_version=11.4
  q : Outport
  wires:
  Register0/1 -> Register1/1
  Register1/1 -> Register2/1
  Register2/1 -> Register3/1
  d/1 -> Register0/1
  Register3/1 -> q/1

== system pfb_float_4k_sim/pipeline56 ==
  d : Inport
  Register{0..3} (4 alike) : xbsIndex_r4/Register  init=0  rst=off  en=off  dbl_ovrd=off  block_type=register
      Register0 settings: block_version=11.4
      Register1 settings: block_version=11.4
      Register2 settings: block_version=11.4
  q : Outport
  wires:
  Register0/1 -> Register1/1
  Register1/1 -> Register2/1
  Register2/1 -> Register3/1
  d/1 -> Register0/1
  Register3/1 -> q/1

== system pfb_float_4k_sim/pipeline57 ==
  d : Inport
  Register0 : xbsIndex_r4/Register  init=0  rst=off  en=off  dbl_ovrd=off  block_type=register
      settings: block_version=11.4
  Register1 : xbsIndex_r4/Register  init=0  rst=off  en=off  dbl_ovrd=off  block_type=register
      settings: block_version=11.4
  q : Outport
  wires:
  Register0/1 -> Register1/1
  d/1 -> Register0/1
  Register1/1 -> q/1

== system pfb_float_4k_sim/pipeline58 ==
  d : Inport
  Register0 : xbsIndex_r4/Register  init=0  rst=off  en=off  dbl_ovrd=off  block_type=register
      settings: block_version=11.4
  Register1 : xbsIndex_r4/Register  init=0  rst=off  en=off  dbl_ovrd=off  block_type=register
      settings: block_version=11.4
  q : Outport
  wires:
  Register0/1 -> Register1/1
  d/1 -> Register0/1
  Register1/1 -> q/1

== system pfb_float_4k_sim/pipeline59 ==
  d : Inport
  Register{0..3} (4 alike) : xbsIndex_r4/Register  init=0  rst=off  en=off  dbl_ovrd=off  block_type=register
      Register0 settings: block_version=11.4
      Register1 settings: block_version=11.4
      Register2 settings: block_version=11.4
  q : Outport
  wires:
  Register0/1 -> Register1/1
  Register1/1 -> Register2/1
  Register2/1 -> Register3/1
  d/1 -> Register0/1
  Register3/1 -> q/1

== system pfb_float_4k_sim/pipeline6 ==
  d : Inport
  Register0 : xbsIndex_r4/Register  init=0  rst=off  en=off  dbl_ovrd=off  block_type=register
      settings: block_version=11.4
  Register1 : xbsIndex_r4/Register  init=0  rst=off  en=off  dbl_ovrd=off  block_type=register
      settings: block_version=11.4
  q : Outport
  wires:
  Register0/1 -> Register1/1
  d/1 -> Register0/1
  Register1/1 -> q/1

== system pfb_float_4k_sim/pipeline60 ==
  d : Inport
  Register0 : xbsIndex_r4/Register  init=0  rst=off  en=off  dbl_ovrd=off  block_type=register
      settings: block_version=11.4
  Register1 : xbsIndex_r4/Register  init=0  rst=off  en=off  dbl_ovrd=off  block_type=register
      settings: block_version=11.4
  q : Outport
  wires:
  Register0/1 -> Register1/1
  d/1 -> Register0/1
  Register1/1 -> q/1

== system pfb_float_4k_sim/pipeline61 ==
  d : Inport
  Register{0..3} (4 alike) : xbsIndex_r4/Register  init=0  rst=off  en=off  dbl_ovrd=off  block_type=register
      Register0 settings: block_version=11.4
      Register1 settings: block_version=11.4
      Register2 settings: block_version=11.4
  q : Outport
  wires:
  Register0/1 -> Register1/1
  Register1/1 -> Register2/1
  Register2/1 -> Register3/1
  d/1 -> Register0/1
  Register3/1 -> q/1

== system pfb_float_4k_sim/pipeline62 ==
  d : Inport
  Register{0..3} (4 alike) : xbsIndex_r4/Register  init=0  rst=off  en=off  dbl_ovrd=off  block_type=register
      Register0 settings: block_version=11.4
      Register1 settings: block_version=11.4
      Register2 settings: block_version=11.4
  q : Outport
  wires:
  Register0/1 -> Register1/1
  Register1/1 -> Register2/1
  Register2/1 -> Register3/1
  d/1 -> Register0/1
  Register3/1 -> q/1

== system pfb_float_4k_sim/pipeline63 ==
  d : Inport
  Register0 : xbsIndex_r4/Register  init=0  rst=off  en=off  dbl_ovrd=off  block_type=register
      settings: block_version=11.4
  Register1 : xbsIndex_r4/Register  init=0  rst=off  en=off  dbl_ovrd=off  block_type=register
      settings: block_version=11.4
  q : Outport
  wires:
  Register0/1 -> Register1/1
  d/1 -> Register0/1
  Register1/1 -> q/1

== system pfb_float_4k_sim/pipeline64 ==
  d : Inport
  Register0 : xbsIndex_r4/Register  init=0  rst=off  en=off  dbl_ovrd=off  block_type=register
      settings: block_version=11.4
  Register1 : xbsIndex_r4/Register  init=0  rst=off  en=off  dbl_ovrd=off  block_type=register
      settings: block_version=11.4
  q : Outport
  wires:
  Register0/1 -> Register1/1
  d/1 -> Register0/1
  Register1/1 -> q/1

== system pfb_float_4k_sim/pipeline65 ==
  d : Inport
  Register0 : xbsIndex_r4/Register  init=0  rst=off  en=off  dbl_ovrd=off  block_type=register
      settings: block_version=11.4
  Register1 : xbsIndex_r4/Register  init=0  rst=off  en=off  dbl_ovrd=off  block_type=register
      settings: block_version=11.4
  q : Outport
  wires:
  Register0/1 -> Register1/1
  d/1 -> Register0/1
  Register1/1 -> q/1

== system pfb_float_4k_sim/pipeline66 ==
  d : Inport
  Register0 : xbsIndex_r4/Register  init=0  rst=off  en=off  dbl_ovrd=off  block_type=register
      settings: block_version=11.4
  Register1 : xbsIndex_r4/Register  init=0  rst=off  en=off  dbl_ovrd=off  block_type=register
      settings: block_version=11.4
  q : Outport
  wires:
  Register0/1 -> Register1/1
  d/1 -> Register0/1
  Register1/1 -> q/1

== system pfb_float_4k_sim/pipeline67 ==
  d : Inport
  Register0 : xbsIndex_r4/Register  init=0  rst=off  en=off  dbl_ovrd=off  block_type=register
      settings: block_version=11.4
  Register1 : xbsIndex_r4/Register  init=0  rst=off  en=off  dbl_ovrd=off  block_type=register
      settings: block_version=11.4
  q : Outport
  wires:
  Register0/1 -> Register1/1
  d/1 -> Register0/1
  Register1/1 -> q/1

== system pfb_float_4k_sim/pipeline68 ==
  d : Inport
  Register0 : xbsIndex_r4/Register  init=0  rst=off  en=off  dbl_ovrd=off  block_type=register
      settings: block_version=11.4
  Register1 : xbsIndex_r4/Register  init=0  rst=off  en=off  dbl_ovrd=off  block_type=register
      settings: block_version=11.4
  q : Outport
  wires:
  Register0/1 -> Register1/1
  d/1 -> Register0/1
  Register1/1 -> q/1

== system pfb_float_4k_sim/pipeline69 ==
  d : Inport
  Register0 : xbsIndex_r4/Register  init=0  rst=off  en=off  dbl_ovrd=off  block_type=register
      settings: block_version=11.4
  Register1 : xbsIndex_r4/Register  init=0  rst=off  en=off  dbl_ovrd=off  block_type=register
      settings: block_version=11.4
  q : Outport
  wires:
  Register0/1 -> Register1/1
  d/1 -> Register0/1
  Register1/1 -> q/1

== system pfb_float_4k_sim/pipeline7 ==
  d : Inport
  Register0 : xbsIndex_r4/Register  init=0  rst=off  en=off  dbl_ovrd=off  block_type=register
      settings: block_version=11.4
  Register1 : xbsIndex_r4/Register  init=0  rst=off  en=off  dbl_ovrd=off  block_type=register
      settings: block_version=11.4
  q : Outport
  wires:
  Register0/1 -> Register1/1
  d/1 -> Register0/1
  Register1/1 -> q/1

== system pfb_float_4k_sim/pipeline70 ==
  d : Inport
  Register0 : xbsIndex_r4/Register  init=0  rst=off  en=off  dbl_ovrd=off  block_type=register
      settings: block_version=11.4
  Register1 : xbsIndex_r4/Register  init=0  rst=off  en=off  dbl_ovrd=off  block_type=register
      settings: block_version=11.4
  q : Outport
  wires:
  Register0/1 -> Register1/1
  d/1 -> Register0/1
  Register1/1 -> q/1

== system pfb_float_4k_sim/pipeline71 ==
  d : Inport
  Register0 : xbsIndex_r4/Register  init=0  rst=off  en=off  dbl_ovrd=off  block_type=register
      settings: block_version=11.4
  Register1 : xbsIndex_r4/Register  init=0  rst=off  en=off  dbl_ovrd=off  block_type=register
      settings: block_version=11.4
  q : Outport
  wires:
  Register0/1 -> Register1/1
  d/1 -> Register0/1
  Register1/1 -> q/1
